simulink library "Renault_Library"  (Simulink 9.2)
settings: Solver=ode3  StartTime=0.0  StopTime=10.0  FixedStep=0.020  RelTol=1e-3  EnableMultiTasking=on
undefined symbols (not defined in the file or in its folder): ABC, AssystemProposal, b, chkval, CoeffA_C, CoeffB_C, CoeffMean_C, ConfTime_C, DiagDelay_C, DividePercent, FailureConfActive_C, FallingEdgeDuration_C, HighLevelThresholdVoltage_C, HighLevelVoltage_C, HighLevelVoltageTunable_C, InitValue_C, InputVoltRangeMax_C, InputVoltRangeMin_C, LowActiveContact_C, LowLevelThresholdVoltage_C, LowLevelVoltage_C, MaxOperatingVoltage_C, MinOperatingVoltage_C, mV2V_conversion, OUT1, OutOfRangeHigh_C, OutOfRangeLow_C, PinSupplySaturationMax, PinSupplySaturationMin, Res1Value_C, Res2Value_C, Res3Value_C, RisingEdgeDuration_C, RSA_CANSignalFailMonitoring, SampleTime_C, service14Table, service19Table, service22Table, service24Table, service28Table, service2ETable, service2FTable, service31Table, service87Table, SizeXStateDID2FTable, SizeXTable14, SizeXTable19, SizeXTable22, SizeXTable24, SizeXTable28, SizeXTable2E, SizeXTable2F, SizeXTable31, SizeXTable87, SizeYStateDID2FTable, SizeYTable14, SizeYTable19, SizeYTable22, SizeYTable24, SizeYTable28 ... (+22)
undefined symbols (not defined in the file): BatteryVoltage_ActivationThreshold_C, FrameStatus_AbsentValue_C, FrameStatus_ConfAbsentValue_C, MemThreshold_C, NAMEOFTHEMODEL_SAMPLE_TIME_CST, NbAbs1_C, NbConfAbs1_C, NbConfPres1_C, NbPres1_C, NOMDUSIGNAL_FaultConfTime_C, NOMDUSIGNAL_FaultRehabTime_C, NOMDUSIGNAL_switchvalue_C, NOMDUSIGNAL_unavailablevalue_C, PeriodFrame1_C, ResetTime_C, WakeUpTime_C
libraries: $bdroot x112, simulink x75
(part 3 of 32)

== system Renault_Library/Complex blocks/Signal Routing ==
  note: Signal Routing
  note: /!\         DO NOT USE THIS RED BLOCKS ANYMORE          /!\
      |
      |
      |
      |
      |
      |
      |
      |
      |
      |
      |
      |
      |
      |
      |
      |
      |
      |
      |
      |
      |
      |
      |
      |
      |
      |
      |
      | .
  RSA_DataTypeDuplicate : Subsystem  Tag=[LIB]  mask(RSA_DataTypeDuplicate){NoIn=3}
      mask prompts: NoIn "No Of Inputs"
      mask help:
      | Force all inputs to have the exact same data type.
      |
      | Version : 01.02
      settings: AttributesFormatString=%<MaskType> : Ver : 01.02\nNo Of Inputs %<NoIn>  CopyFcn=set(gcbh,'linkstatus','none');
      mask Help: eval(['open([','''\\F-khepri\shared\DIM-DCMAP-CGMP\66161\Shared_MatLab\Tools_DCMAP\Common_Library_MBDR\Library\Common_Library\Help\RLV_RSA_DataTypeDuplicate_Spec_V1.02.pdf''','])'])
      mask Callback NoIn:
      | f= str2num(get_param(gcb, 'NoIn'));
      | gcb;
      | d1=find_system(gcb,'LookUnderMasks','all','BlockType','Inport');
      | set_param([gcb '/Mux'],'Inputs',num2str(f));
      | if f ~= length(d1)
      | for i = 1:length(d1)
      |     b=i*50;
      |     a=150;
      |     hanblk=get_param(d1{i},'handle');
      |     blkDet=get(hanblk);
      |     pos=blkDet.PortConnectivity(1).Position;
      |     delete_line(gcb,pos);
      | delete_block(d1{i});
      | end
      | 
      | for i = 1:f
      |     b=i*50;
      |     a=150;
      |     append = num2str(i);
      |     append=strcat('/In',append);
      | 	PortName = strcat(gcb,append);
      | %     PortNameTer = strcat(PortName,'Ter');
      |     add_block('simulink/Ports & Subsystems/In1',PortName,'Position',[a-60 b-5 a-40 b+8])
      |     set_param(PortName,'ShowName','On')
      |     set_param(PortName, 'AttributesFormatString','Type : %<OutDataType>\nScaling : %<OutScaling>')
      |     set_param(PortName, 'Backgroundcolor','green');
      |     add_line(gcb,['In' num2str(i) '/1'],['Mux/' num2str(i)],'Autorouting','On');
      | %     add_block('simulink/Sinks/Terminator',PortNameTer,'Position',[a+50-60 b-5 a+50-40 b+5])
      | %     hanblk=get_param(PortName,'handle');
      | %     blkDet=get(hanblk);
      | %     pos=blkDet.PortConnectivity(1).Position;
      | %     hanblk=get_param(PortNameTer,'handle');
      | %     blkDet=get(hanblk);
      | %     pos1=blkDet.PortConnectivity(1).Position;
      | %     add_line(gcb,[pos;pos1])
      | end
      | end
      mask Display: disp('Same\n DT')
  RSA_DataTypeDuplicate_v01_03 : Subsystem  Tag=[LIB]  mask(RSA_DataTypeDuplicate){NoIn=3}
      mask prompts: NoIn "No Of Inputs"
      mask help:
      | Force all inputs to have the exact same data type.
      |
      | Version : 01.03
      mask init:
      | f= str2num(get_param(gcb, 'NoIn'));
      | gcb;
      | d1=find_system(gcb,'LookUnderMasks','all','Followlinks','on','BlockType','Inport');
      | set_param([gcb '/Mux'],'Inputs',num2str(f));
      | if f ~= length(d1)
      | for i = 1:length(d1)
      |     b=i*50;
      |     a=150;
      |     hanblk=get_param(d1{i},'handle');
      |     blkDet=get(hanblk);
      |     pos=blkDet.PortConnectivity(1).Position;
      |     delete_line(gcb,pos);
      | delete_block(d1{i});
      | end
      | 
      | for i = 1:f
      |     b=i*50;
      |     a=150;
      |     append = num2str(i);
      |     append=strcat('/In',append);
      | 	PortName = strcat(gcb,append);
      |     add_block('built-in/Inport',PortName,'Position',[a-60 b-5 a-40 b+8])
      |     set_param(PortName,'ShowName','On')
      |     set_param(PortName, 'Backgroundcolor','green'); 
      |     add_line(gcb,['In' num2str(i) '/1'],['Mux/' num2str(i)],'Autorouting','On');
      | end
      | end
      settings: AttributesFormatString=%<MaskType> : Ver : 01.03\nNo Of Inputs %<NoIn>
      mask Help: eval(['open([','''\\F-khepri\shared\DIM-DCMAP-CGMP\66161\Shared_MatLab\Tools_DCMAP\Common_Library_MBDR\Library\Common_Library\Help\RLV_RSA_DataTypeDuplicate_Spec_V1.03.pdf''','])'])
      mask Display: disp('Same\n DT')
  RSA_SlopeSwitch2Inputs : Subsystem  Tag=[LIB]  mask(RSA_SlopeSwitch2Inputs){st=0.1}
      mask prompts: st "Sample Time used for calculation, should not be inherited (-1)."
      mask help:
      | This block is a Multiple Port Slope Switch.
      | Depending on the value of input Switch_Cmd (from 0), the block outputs the corresponding input signal.
      | For Switch_Cmd = 0, Output is In0, for Switch_Cmd = 1, Output is In1, and so on...
      |
      | Nevertheless, for each switch between two input signals, a slope is activated from the first input signal to the second input signal.
      | The value of this slope is given by Pos_Slope if the input signal to reach is over the initial input signal and by Neg_Slope if the input signal to reach is under.
      | Pos_Slope and Neg_Slope are respectively the positive and negative slope parameters per second.
      |
      | The output Slope_On indicates if a transition is active (Slope_On at true) or not (Slope_On at false).
      |
      | In order to avoid the overflow, the maximum/ minimun range for the inputs Pos_Slope and Neg_Slope shall be choosen as:
      | max range(Pos_Slope)=( max range(data type(In)) – max(In));
      | min range(Neg_Slope)=( min range(data type(Input)) – min(Input));
      |
      | Version : 01.02
      mask init:
      | SS2ININI = 0;
      | STHCMD = 1;
      settings: AttributesFormatString=%<MaskType> : Ver : 01.02
      mask Help: eval(['open([','''\\F-khepri\shared\DIM-DCMAP-CGMP\66161\Shared_MatLab\Tools_DCMAP\Common_Library_MBDR\Library\Common_Library\Help\RLV_RSA_SlopeSwitch2Inputs_Spec_V1.02.pdf''','])'])
      mask Display:
      | color ('blue')
      | disp('SLOPE SWITCH\n\n 2 INPUTS')

== system Renault_Library/Complex blocks/Timers & Counters ==
  note: Timer & Counter
  note: /!\         DO NOT USE THIS RED BLOCKS ANYMORE               /!\
      |
      |
      |
      |
      |
      |
      |
      |
      |
      |
      |
      |
      |
      |
      |
      |
      |
      |
      |
      |
      |
      |
      |
      |
      |
      |
      |
      |
      |
      |
      |
      |
      |
      |
      |
      |
      |
      |
      |
      |
      |
      |
      |
      |
      |
      |
      |
      |
      |
      |
      |
      |
      |
      |
      |
      |
      |
      |
      |
      |
      |
      |
      |
      | .
  RSA_IncAndDecCounter : Subsystem  Tag=[LIB]  mask(RSA_IncAndDecCounter){}
      mask help:
      | Initially the value of “INIT_COUNTER” is set in the “COUNTER_OUTPUT” as initial value. If the “INC_COUNTER” is 1,
      | “COUNTER_OUTPUT” increases with the value of the “COUNTER_ STEP”. If the “DEC_COUNTER” is 1 and “INC_COUNTER” is 0,
      | the “COUNTER_OUTPUT” decreases with the value of the “COUNTER_ STEP”.
      |
      | The “COUNTER_OUTPUT” stops increasing or decreasing when it reaches to "MAX_COUNTER" and " MIN_COUNTER ".
      | If the “RESET_COUNTER” is 1, “COUNTER_OUTPUT” is reset to the value zero.
      |
      | Version : 01.00
      settings: AttributesFormatString=%<MaskType> : Ver : 01.00
      mask Help: eval(['open([','''\\F-khepri\shared\DIM-DCMAP-CGMP\66161\Shared_MatLab\Tools_DCMAP\Common_Library_MBDR\Library\Common_Library\Help\RLV_RSA_IncAndDecCounter_Spec_V1.00.pdf''','])'])
  RSA_IncAndDecCounter_V01_01 : Subsystem  Tag=[LIB]  mask(RSA_IncAndDecCounter){}
      mask help:
      | Initially the value of “INIT_COUNTER” is set in the “COUNTER_OUTPUT” as initial value. If the “INC_COUNTER” is 1,
      | “COUNTER_OUTPUT” increases with the value of the “COUNTER_ STEP”. If the “DEC_COUNTER” is 1 and “INC_COUNTER” is 0,
      | the “COUNTER_OUTPUT” decreases with the value of the “COUNTER_ STEP”.
      |
      | The “COUNTER_OUTPUT” stops increasing or decreasing when it reaches to "MAX_COUNTER" and " MIN_COUNTER ".
      | If the “RESET_COUNTER” is 1, “COUNTER_OUTPUT” is reset to the value zero.
      |
      | Version : 01.01
      mask init:
      | CONS_ZERO=0;
      | CONS_ONE=1;
      | CONS_TWO=2;
      | CONS_THREE=3;
      settings: AttributesFormatString=%<MaskType> : Ver : 01.01
      mask Help: eval(['open([','''\\F-khepri\shared\DIM-DCMAP-CGMP\66161\Shared_MatLab\Tools_DCMAP\Common_Library_MBDR\Library\Common_Library\Help\RLV_RSA_IncAndDecCounter_Spec_V1.01.pdf''','])'])
  RSA_Timer : Subsystem  Tag=[LIB]  mask(RSA_Timer){timeoutinit=0, initinit=0, st=0.1}
      mask prompts: timeoutinit "Timeout output initialisation"; initinit "Init input initialisation"; st "Sample Time used for calculation, should not be inherited (-1)."
      mask help:
      | This block starts a timer and generates a timeout signal when the required duration has elapsed.
      | The duration in seconds is set on rising edge 'Init' signal  and counting phase begins.
      | Output 'Timer' gives the remaining time in seconds, that must pass before generating the 'Timeout' signal.
      | If the 'Init' signal is true after the power supply, and if the timer must start, set init input initialisation to 0, else set it to 1.
      | If the 'Timeout' output must start at true, set timeout output initialisation to 1, else set it to 0.
      |
      | Version : 01.03
      mask init:
      | TIM1INI = 0;
      | Least_value = 0.000000001;
      settings: AttributesFormatString=%<MaskType> : Ver : 01.03\nTimeout output initialisation=%<timeoutinit>\nInit input initialisation=%<initinit>
      mask Help: eval(['open([','''\\F-khepri\shared\DIM-DCMAP-CGMP\66161\Shared_MatLab\Tools_DCMAP\Common_Library_MBDR\Library\Common_Library\Help\RLV_RSA_Timer_Spec_V1.03.pdf''','])'])
      mask Display:
      | plot([0.1 0.15 0.15 0.2 0.2 0.35],[0.67 0.67 0.9 0.9 0.67 0.67]);
      | plot([0.45 0.5 0.5 0.7 0.9],[0.05 0.05 0.3 0.05 0.05])
      | plot([0.45 0.7 0.7 0.9],[0.67 0.67 0.9 0.9])
  RSA_Timer1 : Subsystem  Tag=[LIB]  mask(RSA_Timer1){timeoutinit=0, initinit=0}
      mask prompts: timeoutinit "Timeout output initialisation"; initinit "Init input initialisation"
      mask help:
      | The function of the timer block is to provide "Timeout" signal after the set "Duration" is elapsed by "Timestep" when rising triggered transition by "Init" flag at input. The output value of "Duration" is also available at "Timer" output signal according to elapsed time as set via "Timestep" input signal.
      |
      | Version : 01.03
      mask init:
      | TIM1INI = 0;
      settings: AttributesFormatString=%<MaskType> : Ver : 01.03\nTimeout output initialisation=%<timeoutinit>\nInit input initialisation=%<initinit>
      mask Help: eval(['open([','''\\F-khepri\shared\DIM-DCMAP-CGMP\66161\Shared_MatLab\Tools_DCMAP\Common_Library_MBDR\Library\Common_Library\Help\RLV_RSA_Timer1_Spec_V1.03.pdf''','])'])
      mask Display:
      | plot([0.1 0.15 0.15 0.2 0.2 0.35],[0.67 0.67 0.9 0.9 0.67 0.67]);
      | plot([0.45 0.5 0.5 0.7 0.9],[0.05 0.05 0.3 0.05 0.05])
      | plot([0.45 0.7 0.7 0.9],[0.67 0.67 0.9 0.9])
  RSA_Timer1_v01_04 : Subsystem  Tag=[LIB]  mask(RSA_Timer1){timeoutinit=0, initinit=0}
      mask prompts: timeoutinit "Timeout output initialisation"; initinit "Init input initialisation"
      mask help:
      | The function of the timer block is to provide "Timeout" signal after the set "Duration" is elapsed by "Timestep" when rising triggered transition by "Init" flag at input. The output value of "Duration" is also available at "Timer" output signal according to elapsed time as set via "Timestep" input signal.
      |
      | Version : 01.04
      mask init:
      | TIM1INI = 0;
      settings: AttributesFormatString=%<MaskType> : Ver : 01.04\nTimeout output initialisation=%<timeoutinit>\nInit input initialisation=%<initinit>
      mask Help: eval(['open([','''\\F-khepri\shared\DIM-DCMAP-CGMP\66161\Shared_MatLab\Tools_DCMAP\Common_Library_MBDR\Library\Common_Library\Help\RLV_RSA_Timer1_Spec_V1.04.pdf''','])'])
      mask Display:
      | plot([0.1 0.15 0.15 0.2 0.2 0.35],[0.67 0.67 0.9 0.9 0.67 0.67]);
      | plot([0.45 0.5 0.5 0.7 0.9],[0.05 0.05 0.3 0.05 0.05])
      | plot([0.45 0.7 0.7 0.9],[0.67 0.67 0.9 0.9])
  RSA_Timer1_v01_05 : Subsystem  Tag=[LIB]  mask(RSA_Timer1){timeoutinit=0, initinit=0}
      mask prompts: timeoutinit "Timeout output initialisation"; initinit "Init input initialisation"
      mask help:
      | The function of the timer block is to provide "Timeout" signal after the set "Duration" is elapsed by "Timestep" when rising triggered transition by "Init" flag at input. The output value of "Duration" is also available at "Timer" output signal according to elapsed time as set via "Timestep" input signal.
      |
      | Note:
      | The type of the input "Duration" should not be inherited. The type should be mentioned in the input "Duration"
      |
      | Version : 01.05
      mask init:
      | TIM1INI = 0;
      settings: AttributesFormatString=%<MaskType> : Ver : 01.05\nTimeout output initialisation=%<timeoutinit>\nInit input initialisation=%<initinit>
      mask Help: eval(['open([','''\\F-khepri\shared\DIM-DCMAP-CGMP\66161\Shared_MatLab\Tools_DCMAP\Common_Library_MBDR\Library\Common_Library\Help\RLV_RSA_Timer1_Spec_V1.05.pdf''','])'])
      mask Display:
      | plot([0.1 0.15 0.15 0.2 0.2 0.35],[0.67 0.67 0.9 0.9 0.67 0.67]);
      | plot([0.45 0.5 0.5 0.7 0.9],[0.05 0.05 0.3 0.05 0.05])
      | plot([0.45 0.7 0.7 0.9],[0.67 0.67 0.9 0.9])
  RSA_Timer1_v01_06 : Subsystem  Tag=[LIB]  mask(RSA_Timer1){timeoutinit=0, initinit=0}
      mask prompts: timeoutinit "Timeout output initialisation"; initinit "Init input initialisation"
      mask help:
      | When the input2 of the block ("Init") is rising to 1, the following will be happening
      |     1. The output ("Timesout") will be Rising to 1 (TRUE) after a Delay (Duration/Timestep).
      |     2. The output "Timer" will be set with "Duration" Value and start Decrementing by SampleTime.
      |
      | When the input3 of the block ("Timestep") is falling to 0, the following will be happening
      |     1. Block will be reset to Initial State (Timeout = 0 and Timer = 0)
      |
      | Initializations:
      | - Output initialization = beginning state of Output signal.
      | - Input initialization = Used to determine rising or falling edge at the first simulation step.
      |     0 - Block will detect Edge Rising at the first simulation step itself
      |     1 - Block will detect Edge Rising at the first simulation step
      |
      | Warning: Please note that TotalDelay = (Duration/Timestep)
      |
      | Note:
      | 1. Negative Values are not allowed as an inputs (Duration,Timestep) to the block
      | 2. The Block's inputs (Duration,Timestep) must have Same Datatype
      | 3. The Block's inputs (Duration,Timestep) should not be Zero value when there is a Rising Edge of input (Init)
      |
      | Version : 01.06
      mask init:
      | TIM1INI = 0;
      | Boosting_Decimal=1000;
      | Boosting_Whole=1;
      | CONS_ONE=1;
      | CONS_ZERO=0;
      settings: AttributesFormatString=%<MaskType> : Ver : 01.06\nTimeout output initialisation=%<timeoutinit>\nInit input initialisation=%<initinit>
      mask Help: eval(['open([','''\\F-khepri\shared\DIM-DCMAP-CGMP\66161\Shared_MatLab\Tools_DCMAP\Common_Library_MBDR\Library\Common_Library\Help\RLV_RSA_Timer1_Spec_V1.06.pdf''','])'])
      mask Display:
      | plot([0.1 0.15 0.15 0.2 0.2 0.35],[0.67 0.67 0.9 0.9 0.67 0.67]);
      | plot([0.45 0.5 0.5 0.7 0.9],[0.05 0.05 0.3 0.05 0.05])
      | plot([0.45 0.7 0.7 0.9],[0.67 0.67 0.9 0.9])
  RSA_Timer_Conversion : Subsystem  Tag=[LIB]  mask(RSA_Timer_Conversion){SAMPLE_TIME_CST=NAMEOFTHEMODEL_SAMPLE_TIME_CST}
      mask prompts: SAMPLE_TIME_CST "Sample time :"
      mask help:
      | Timer Conversion block is to adapt a calibration value to output depending on a constant in block mask.
      |
      | Note:
      | The sample time constant "MODEL_NAME_SAMPLE_TIME_CST" should be defined in the workspace and the value must not be 0 or -1.
      |
      | Version : 01.00
      mask init:
      | ZERO_CST = 0;
      settings: AttributesFormatString=%<MaskType> : Ver : 01.00
      mask Help: eval(['open([','''\\F-khepri\shared\DIM-DCMAP-CGMP\66161\Shared_MatLab\Tools_DCMAP\Common_Library_MBDR\Library\Common_Library\Help\RLV_RSA_Timer_Conversion_Spec_V1.00.pdf''','])'])
  RSA_Timer_Conversion1 : Subsystem  Tag=[LIB]  mask(RSA_Timer_Conversion){SAMPLE_TIME_CST=NAMEOFTHEMODEL_SAMPLE_TIME_CST}
      mask prompts: SAMPLE_TIME_CST "Sample time :"
      mask help:
      | Timer Conversion block is to adapt a calibration value to output depending on a constant in block mask.
      |
      | Note:
      | 1) The sample time constant "MODEL_NAME_SAMPLE_TIME_CST" should be defined in the workspace and the value must not be 0 or -1.
      | 2) If the sample time is zero, then input is passed without any conversion.
      | 3) If the Sample Time in the Model where the Block is used, is not given any –ve or Zero Value, then this will lead to Model Coverage drops
      |
      | Version : 01.02
      mask init:
      | ZERO_CST = 0;
      settings: AttributesFormatString=|
      | %<MaskType> : Ver : 01.02
      | Sample Time : %<SAMPLE_TIME_CST>
      mask Help: eval(['open([','''\\F-khepri\shared\DIM-DCMAP-CGMP\66161\Shared_MatLab\Tools_DCMAP\Common_Library_MBDR\Library\Common_Library\Help\RLV_RSA_Timer_Conversion_Spec_V1.02.pdf''','])'])
  RSA_Timer_Conversion_v01_01 : Subsystem  Tag=[LIB]  mask(RSA_Timer_Conversion){SAMPLE_TIME_CST=NAMEOFTHEMODEL_SAMPLE_TIME_CST}
      mask prompts: SAMPLE_TIME_CST "Sample time :"
      mask help:
      | Timer Conversion block is to adapt a calibration value to output depending on a constant in block mask.
      |
      | Note:
      | The sample time constant "MODEL_NAME_SAMPLE_TIME_CST" shuold be defined in the workspace and the value must not be 0 or -1.
      |
      | Version : 01.01
      mask init:
      | ZERO_CST = 0;
      settings: AttributesFormatString=%<MaskType> : Ver : 01.01
      mask Help: eval(['open([','''\\F-khepri\shared\DIM-DCMAP-CGMP\66161\Shared_MatLab\Tools_DCMAP\Common_Library_MBDR\Library\Common_Library\Help\RLV_RSA_Timer_Conversion_Spec_V1.01.pdf''','])'])
  RSA_Timer_v01_04 : Subsystem  Tag=[LIB]  mask(RSA_Timer){timeoutinit=0, initinit=0, st=0.1}
      mask prompts: timeoutinit "Timeout output initialisation"; initinit "Init input initialisation"; st "Sample Time used for calculation, should not be inherited (-1)."
      mask help:
      | This block starts a timer and generates a timeout signal when the required duration has elapsed.
      | The duration in seconds is set on rising edge 'Init' signal  and counting phase begins.
      | Output 'Timer' gives the remaining time in seconds, that must pass before generating the 'Timeout' signal.
      | If the 'Init' signal is true after the power supply, and if the timer must start, set init input initialisation to 0, else set it to 1.
      | If the 'Timeout' output must start at true, set timeout output initialisation to 1, else set it to 0.
      |
      | Version : 01.04
      mask init:
      | TIM1INI = 0;
      | Least_value = 0.000000001;
      settings: AttributesFormatString=%<MaskType> : Ver : 01.04\nTimeout output initialisation=%<timeoutinit>\nInit input initialisation=%<initinit>
      mask Help: eval(['open([','''\\F-khepri\shared\DIM-DCMAP-CGMP\66161\Shared_MatLab\Tools_DCMAP\Common_Library_MBDR\Library\Common_Library\Help\RLV_RSA_Timer_Spec_V1.04.pdf''','])'])
      mask Display:
      | plot([0.1 0.15 0.15 0.2 0.2 0.35],[0.67 0.67 0.9 0.9 0.67 0.67]);
      | plot([0.45 0.5 0.5 0.7 0.9],[0.05 0.05 0.3 0.05 0.05])
      | plot([0.45 0.7 0.7 0.9],[0.67 0.67 0.9 0.9])
  RSA_Timer_v01_05 : Subsystem  Tag=[LIB]  mask(RSA_Timer){timeoutinit=0, initinit=0, st=1}
      mask prompts: timeoutinit "Timeout output initialisation"; initinit "Init input initialisation"; st "Sample Time used for calculation, should not be inherited (-1)."
      mask help:
      | This block starts a timer and generates a timeout signal when the required duration has elapsed.
      | The duration in seconds is set on rising edge 'Init' signal  and counting phase begins.
      | Output 'Timer' gives the remaining time in seconds, that must pass before generating the 'Timeout' signal.
      | If the 'Init' signal is true after the power supply, and if the timer must start, set init input initialisation to 0, else set it to 1.
      | If the 'Timeout' output must start at true, set timeout output initialisation to 1, else set it to 0.
      |
      | Note:
      | The type of the input "Duration" should not be inherited from anywhere, it should be mentioned the type of input.
      |
      | Version : 01.05
      mask init:
      | TIM1INI = 0;
      | Least_value = 0.000000001;
      settings: AttributesFormatString=%<MaskType> : Ver : 01.05\nTimeout output initialisation=%<timeoutinit>\nInit input initialisation=%<initinit>
      mask Help: eval(['open([','''\\F-khepri\shared\DIM-DCMAP-CGMP\66161\Shared_MatLab\Tools_DCMAP\Common_Library_MBDR\Library\Common_Library\Help\RLV_RSA_Timer_Spec_V1.05.pdf''','])'])
      mask Display:
      | plot([0.1 0.15 0.15 0.2 0.2 0.35],[0.67 0.67 0.9 0.9 0.67 0.67]);
      | plot([0.45 0.5 0.5 0.7 0.9],[0.05 0.05 0.3 0.05 0.05])
      | plot([0.45 0.7 0.7 0.9],[0.67 0.67 0.9 0.9])
  RSA_Timer_v01_06 : Subsystem  Tag=[LIB]  mask(RSA_Timer){timeoutinit=0, initinit=0, st=0.01}
      mask prompts: timeoutinit "Timeout output initialisation"; initinit "Init input initialisation"; st "Sample Time used for calculation, should not be inherited (-1)."
      mask help:
      | This block starts a timer and generates a timeout signal when the required duration has elapsed.
      | The duration in seconds is set on rising edge 'Init' signal  and counting phase begins.
      | Output 'Timer' gives the remaining time in seconds, that must pass before generating the 'Timeout' signal.
      | If the 'Init' signal is true after the power supply, and if the timer must start, set init input initialisation to 0, else set it to 1.
      | If the 'Timeout' output must start at true, set timeout output initialisation to 1, else set it to 0.
      |
      | Note:
      | The type of the input "Duration" should not be inherited from anywhere, it should be mentioned the type of input.
      |
      | Version : 01.06
      mask init:
      | TIM1INI = 0;
      | Least_value = 0.000000001;
      settings: AttributesFormatString=%<MaskType> : Ver : 01.06\nTimeout output initialisation=%<timeoutinit>\nInit input initialisation=%<initinit>
      mask Help: eval(['open([','''\\F-khepri\shared\DIM-DCMAP-CGMP\66161\Shared_MatLab\Tools_DCMAP\Common_Library_MBDR\Library\Common_Library\Help\RLV_RSA_Timer_Spec_V1.06.pdf''','])'])
      mask Display:
      | plot([0.1 0.15 0.15 0.2 0.2 0.35],[0.67 0.67 0.9 0.9 0.67 0.67]);
      | plot([0.45 0.5 0.5 0.7 0.9],[0.05 0.05 0.3 0.05 0.05])
      | plot([0.45 0.7 0.7 0.9],[0.67 0.67 0.9 0.9])
  RSA_Timer_v01_07 : Subsystem  Tag=[LIB]  mask(RSA_Timer){timeoutinit=0, initinit=0, st=0.1, SampleDT=double}
      mask prompts: timeoutinit "Timeout output initialisation"; initinit "Init input initialisation"; st "Sample Time used for calculation, should not be inherited (-1)."; SampleDT "DataType for Sample Time"
      mask help:
      | When the input1 of the block ("Init") is rising to 1, the following will be happening
      |     1. The output "Timer" will be set with "Duration" Value and start Decrementing by SampleTime.
      |     2. The output ("Timeout") will be Rising to 1 (TRUE) after a Delay (Duration/SampleTime).
      |
      | When the input1 of the block ("Init") is falling to 0, the following will be happening
      |     1. Block will be reset to Initial State (delay_out = 0 and Timer =0)
      |
      | WARNING: Please note that Total Delay  = (Duration/SampleTime).
      |
      | Version : 01.07
      mask init:
      | TIM1INI = 0;
      | fix_flag = ~isempty(regexp(get(gcbh,'SampleDT'),'^single$|^double$'));
      | if fix_flag
      |     Boosting_Factor = 1000;
      |     Constant_DT = get(gcbh,'SampleDT');
      | else
      |     Boosting_Factor = 1;
      |     Constant_DT = 'uint8';
      | end
      settings: AttributesFormatString=|
      | %<MaskType> : Ver : 01.07\nTimeout output initialisation=%<timeoutinit>\nInit input initialisation=%<initinit>
      | Sample Time : %<st>
      | Sample Time DataType :%<SampleDT>
      mask Help: eval(['open([','''\\F-khepri\shared\DIM-DCMAP-CGMP\66161\Shared_MatLab\Tools_DCMAP\Common_Library_MBDR\Library\Common_Library\Help\RLV_RSA_Timer_Spec_V1.07.pdf''','])'])
      mask Display:
      | plot([0.1 0.15 0.15 0.2 0.2 0.35],[0.67 0.67 0.9 0.9 0.67 0.67]);
      | plot([0.45 0.5 0.5 0.7 0.9],[0.05 0.05 0.3 0.05 0.05])
      | plot([0.45 0.7 0.7 0.9],[0.67 0.67 0.9 0.9])
  RSA_TurnOffDelay2 : Subsystem  Tag=[LIB]  mask(RSA_TurnOffDelay2){Ts=0.1}
      mask prompts: Ts "Sample Time used for calculation, should not be inherited (-1)."
      mask help:
      | TurnOff Delay block will delay the turnoff time 'out' with respect to the given delay time 'dly'.
      |
      | Version : 01.02
      mask init:
      | INIVAL = 0;
      settings: AttributesFormatString=%<MaskType> : Ver : 01.02
      mask Help:
      | TurnOff Delay block will delay the turnoff time 'out' with respect to the given delay time 'dly'.
      | 
      | Version : 01.02
      mask Display:
      | plot([-1.05,12.05],[0.97,0.97]*11);
      | plot([0  1  1  2  2  4  4  7  7  9  9 11],...
      | 	 [10 10 8  8 10 10  8  8  8  8 10 10]);
      | plot([0  1  1  2  2  4  4  7  7  9  9 11],...
      | 	 [4  4  7  6  6  6  7  4  4  4  4  4]);
      | plot([0  1  1  2  2  4  4  7  7  9  9 11],...
      | 	 [3  3  3  3  3  3  3  3  1  1  3  3]);
      | plot(-1,0); plot(12,11);
      | text(4.5,9,'in');
      | text(4.5,5,'ctr');
      | text(4.5,2,'out');
  RSA_TurnOffDelay2_v01_03 : Subsystem  Tag=[LIB]  mask(RSA_TurnOffDelay2){Ts=0.1}
      mask prompts: Ts "Sample Time used for calculation, should not be inherited (-1)."
      mask help:
      | TurnOff Delay block will delay the turnoff time 'out' with respect to the given delay time 'dly'.
      |
      | Version : 01.03
      mask init:
      | INIVAL = 0;
      settings: AttributesFormatString=%<MaskType> : Ver : 01.03
      mask Help: eval(['open([','''\\F-khepri\shared\DIM-DCMAP-CGMP\66161\Shared_MatLab\Tools_DCMAP\Common_Library_MBDR\Library\Common_Library\Help\RLV_RSA_TurnOffDelay2_Spec_V1.03.pdf''','])'])
      mask Display:
      | plot([-1.05,12.05],[0.97,0.97]*11);
      | plot([0  1  1  2  2  4  4  7  7  9  9 11],...
      | 	 [10 10 8  8 10 10  8  8  8  8 10 10]);
      | plot([0  1  1  2  2  4  4  7  7  9  9 11],...
      | 	 [4  4  7  6  6  6  7  4  4  4  4  4]);
      | plot([0  1  1  2  2  4  4  7  7  9  9 11],...
      | 	 [3  3  3  3  3  3  3  3  1  1  3  3]);
      | plot(-1,0); plot(12,11);
      | text(4.5,9,'in');
      | text(4.5,5,'ctr');
      | text(4.5,2,'out');
  RSA_TurnOffDelay2_v01_05 : Subsystem  Tag=[LIB]  mask(RSA_TurnOffDelay2){Ts=1}
      mask prompts: Ts "Sample Time used for calculation, should not be inherited (-1)."
      mask help:
      | TurnOff Delay block will delay the turnoff time 'out' with respect to the given delay time 'dly'.
      |
      | Note:
      | The type of the input "dly" should not be inherited from anywhere, it should be defined with any type.
      | Version : 01.05
      mask init:
      | INIVAL = 0;
      settings: AttributesFormatString=%<MaskType> : Ver : 01.05
      mask Help: eval(['open([','''\\F-khepri\shared\DIM-DCMAP-CGMP\66161\Shared_MatLab\Tools_DCMAP\Common_Library_MBDR\Library\Common_Library\Help\RLV_RSA_TurnOffDelay2_Spec_V1.05.pdf''','])'])
      mask Display:
      | plot([-1.05,12.05],[0.97,0.97]*11);
      | plot([0  1  1  2  2  4  4  7  7  9  9 11],...
      | 	 [10 10 8  8 10 10  8  8  8  8 10 10]);
      | plot([0  1  1  2  2  4  4  7  7  9  9 11],...
      | 	 [4  4  7  6  6  6  7  4  4  4  4  4]);
      | plot([0  1  1  2  2  4  4  7  7  9  9 11],...
      | 	 [3  3  3  3  3  3  3  3  1  1  3  3]);
      | plot(-1,0); plot(12,11);
      | text(4.5,9,'in');
      | text(4.5,5,'ctr');
      | text(4.5,2,'out');
  RSA_TurnOffDelay2_v01_06 : Subsystem  Tag=[LIB]  mask(RSA_TurnOffDelay2){Ts=0.1, SampleDT=double}
      mask prompts: Ts "Sample Time used for calculation, should not be inherited (-1)."; SampleDT "DataType for Sample Time"
      mask help:
      | When the input1 of the block ("delay_flag") is falling to 0, the following will be happening
      |     1. The output "Ctr" will be set with "Dly" Value and start Decrementing by SampleTime.
      |     2. The output ("delay_out") will be falling to 0 (FALSE) after a Delay (Dly/SampleTime).
      |
      | When the input1 of the block ("delay_flag") is rising to 1, the following will be happening
      |     1. Block will be reset to Initial State (delay_out = 1 and Ctr =0)
      |
      | WARNING: Please note that Total Delay  = (Dly/SampleTime).
      |
      | NOTE:
      |     1.When fixed data types is given for input ("dly"), both the input ("dly") and sample time (Ts specified in mask) should have the same resolution.
      |     2.When fixed data types is given for input ("dly"), the sample time's data type in the mask should be set as "Uint8".
      |     3.The input "dly" should be set such that it is a multiple of sample time "Ts". Eg. If "dly" is 1 then allowed sample time (Ts) are 0.1,0.2,0.02, etc.,
      |
      | Version : 01.06
      mask init:
      | %check_non_inherited_samp(Ts);
      | INIVAL = 0;
      | fix_flag = ~isempty(regexp(get(gcbh,'SampleDT'),'^single$|^double$'));
      | if fix_flag
      |     Boosting_Factor = 1000;
      |     Constant_DT = get(gcbh,'SampleDT');
      | else
      |     Boosting_Factor = 1;
      |     Constant_DT = 'uint8';
      | end
      settings: AttributesFormatString=|
      | %<MaskType> : Ver : 01.06
      | Sample Time : %<Ts>
      | Sample Time DataType : %<SampleDT>
      mask Help: eval(['open([','''\\F-khepri\shared\DIM-DCMAP-CGMP\66161\Shared_MatLab\Tools_DCMAP\Common_Library_MBDR\Library\Common_Library\Help\RLV_RSA_TurnOffDelay2_Spec_V1.06.pdf''','])'])
      mask Display:
      | plot([-1.05,12.05],[0.97,0.97]*11);
      | plot([0  1  1  2  2  4  4  7  7  9  9 11],...
      | 	 [10 10 8  8 10 10  8  8  8  8 10 10]);
      | plot([0  1  1  2  2  4  4  7  7  9  9 11],...
      | 	 [4  4  7  6  6  6  7  4  4  4  4  4]);
      | plot([0  1  1  2  2  4  4  7  7  9  9 11],...
      | 	 [3  3  3  3  3  3  3  3  1  1  3  3]);
      | plot(-1,0); plot(12,11);
      | text(4.5,9,'dly_flag');
      | text(4.5,5,'ctr');
      | text(4.5,2,'dly_out');
  RSA_TurnOffDelay_v01_00 : Subsystem  Tag=[LIB]  mask(RSA_TurnOffDelay){outINI=TRUE, inINI=FALSE}
      mask prompts: outINI "Output initialization (false or true)"; inINI "Input initialization (false or true)"
      mask help:
      | When the input1 of the block ("signal") is falling to 0, the following will be happening
      |     1. The output "Ctr" will be set with "Dly" Value and start Decrementing by TimeStep.
      |     2. The output ("out") will be falling to 0 (FALSE) after a Delay (DelayTime/ TimeStep).
      |
      | When the input1 of the block ("signal") is rising to 1, the following will be happening
      |     1. Block will be reset to Initial State (out = 1 and Ctr =0)
      |
      | WARNING: Please note that Total Delay  = (DelayTime / TimeStep).
      | Initializations:
      | - Output initialization = beginning state of Output signal.
      |
      | - Input initialization = Used to determine falling edge at the first simulation step.
      |    1 - Block will detect Edge Falling at the first simulation step itself.
      |    0 - Block will not detect Edge Falling at the first simulation step.
      |
      | Warning: Please note that TotalDelay = (Delaytime/Timestep)
      |
      | Note:
      | 1. Negative Values are not allowed as an inputs (Delaytime,Timestep) to the block
      | 2. The Block's inputs (Delaytime,Timestep) must have Same Datatype
      | 3. The Block's inputs (Delaytime,Timestep) should not be Zero value when there is a Falling Edge of input (Signal)
      | Version: 01.00
      mask init:
      | TIM1INI = 0;
      | CONS_ONE =1;
      | CONS_ZERO=0;
      | BOOSTING_FLP=1000;
      | BOOSTING_FXP=1;
      | if inINI == 2
      |     initinit = boolean(1);
      | else
      |     initinit = boolean(0);
      | end
      | if outINI == 2
      |     timeoutinit = boolean(1);
      | else
      |     timeoutinit = boolean(0);
      | end
      | 
      | if outINI == 2
      |     set_param([gcb '/From7'],'gototag','InitOut');
      | else
      |     set_param([gcb '/From7'],'gototag','InitOut_Cond');
      | end
      settings: AttributesFormatString=%<MaskType> : Ver : 01.00\nOutput initialization=%<outINI>\nInput initialization=%<inINI>
      mask Help: eval(['open([','''\\F-khepri\shared\DIM-DCMAP-CGMP\66161\Shared_MatLab\Tools_DCMAP\Common_Library_MBDR\Library\Common_Library\Help\RLV_RSA_TurnOffDelay_Spec_V1.00.pdf''','])'])
      mask TypeOptions outINI: FALSE | TRUE
      mask TypeOptions inINI: FALSE | TRUE
      mask Display:
      | plot([-1.05,12.05],[0.97,0.97]*11);
      | plot([0  1  1  2  2  4  4  7  7  9  9 11],...
      | 	 [10 10 8  8 10 10  8  8  8  8 10 10]);
      | plot([0  1  1  2  2  4  4  7  7  9  9 11],...
      | 	 [4  4  7  6  6  6  7  4  4  4  4  4]);
      | plot([0  1  1  2  2  4  4  7  7  9  9 11],...
      | 	 [3  3  3  3  3  3  3  3  1  1  3  3]);
      | plot(-1,0); plot(12,11);
      | text(4.5,9,'dly_flag');
      | text(4.5,5,'ctr');
      | text(4.5,2,'dly_out');
  RSA_TurnOnDelay : Subsystem  Tag=[LIB]  mask(RSA_TurnOnDelay){timeoutinit=0, initinit=1}
      mask prompts: timeoutinit "Output initialization (0 or 1)"; initinit "Input initialization (0 or 1)"
      mask help:
      | The RSA_TurnOnDelay function applies a boolean input to the rising edge of the input Signal and delay time on the other input port. The output (Out) is a boolean signal changes from 0 to 1 after the specified delay time.
      |
      | Initializations:
      | - Output initialization = beginning state of Output signal.
      | - Input initialization = state of Signal at -t used to determine the presence of a rising or falling edge at the first simulation step.
      |
      | Version : 01.02
      settings: AttributesFormatString=%<MaskType> : Ver : 01.02\nOutput initialization=%<timeoutinit>\nInput initialization=%<initinit>
      mask Help: eval(['open([','''\\F-khepri\shared\DIM-DCMAP-CGMP\66161\Shared_MatLab\Tools_DCMAP\Common_Library_MBDR\Library\Common_Library\Help\RLV_RSA_TurnOnDelay_Spec_V1.02.pdf''','])'])
      mask Display:
      | plot([3 3.5 3.5 4 4 5 5 7.5 7.5 8.5], [5 5 6 6 5 5 6 6 5 5]);
      | plot([3 6.5 6.5 7.5 7.5 8.5], [2 2 3 3 2 2]);
      | plot([3 4.5], [5 6]);
      | plot([3 4.5], [6 5]);
      | text(5,0.4, 'Duration');
      | plot([5 6.5], [1 1]);
      | plot([5.2 5 5.2], [0.8 1 1.2]);
      | plot([6.3 6.5 6.3], [0.8 1 1.2]);
      | plot([5 5],[4.7 1.3]);
      | plot([6.5 6.5],[1.8 1.3]);
      | plot([7.5 7.5],[4.7 3.7]);
      | plot([0], [0]);
      | plot([8], [7]);
  RSA_TurnOnDelay2 : Subsystem  Tag=[LIB]  mask(RSA_TurnOnDelay2){Ts=0.1}
      mask prompts: Ts "Sample Time used for calculation, should not be inherited (-1)."
      mask help:
      | The output follows input state with a delay to turn ON, without any delay to turn OFF
      | WARNING: dly = counting delay in seconds.
      |
      | Version : 01.02
      mask init:
      | %check_non_inherited_samp(Ts);
      | INIVAL = 0;
      settings: AttributesFormatString=%<MaskType> : Ver : 01.02
      mask Help:
      | The output follows input state with a delay to turn ON, without any delay to turn OFF
      | WARNING: dly = counting delay in seconds.
      | 
      | Version : 01.02
      mask Display:
      | plot([-1.05,12.05],[0.97,0.97]*11);
      | plot([0  1  1  2  2  4  4  7  7  9  9 11],...
      | 	 [8  8 10 10  8  8 10 10 10 10  8  8]);
      | plot([0  1  1  2  2  4  4  7  7  9  9 11],...
      | 	 [4  4  7  6  6  6  7  4  4  4  4  4]);
      | plot([0  1  1  2  2  4  4  7  7  9  9 11],...
      | 	 [1  1  1  1  1  1  1  1  3  3  1  1]);
      | plot(-1,0); plot(12,11);
      | text(4.5,9,'in');
      | text(4.5,5,'ctr');
      | text(4.5,2,'out');
  RSA_TurnOnDelay2_v01_03 : Subsystem  Tag=[LIB]  mask(RSA_TurnOnDelay2){Ts=0.1}
      mask prompts: Ts "Sample Time used for calculation, should not be inherited (-1)."
      mask help:
      | The output follows input state with a delay to turn ON, without any delay to turn OFF
      | WARNING: dly = counting delay in seconds.
      |
      | Version : 01.03
      mask init:
      | %check_non_inherited_samp(Ts);
      | INIVAL = 0;
      settings: AttributesFormatString=%<MaskType> : Ver : 01.03
      mask Help: eval(['open([','''\\F-khepri\shared\DIM-DCMAP-CGMP\66161\Shared_MatLab\Tools_DCMAP\Common_Library_MBDR\Library\Common_Library\Help\RLV_RSA_TurnOnDelay2_Spec_V1.03.pdf''','])'])
      mask Display:
      | plot([-1.05,12.05],[0.97,0.97]*11);
      | plot([0  1  1  2  2  4  4  7  7  9  9 11],...
      | 	 [8  8 10 10  8  8 10 10 10 10  8  8]);
      | plot([0  1  1  2  2  4  4  7  7  9  9 11],...
      | 	 [4  4  7  6  6  6  7  4  4  4  4  4]);
      | plot([0  1  1  2  2  4  4  7  7  9  9 11],...
      | 	 [1  1  1  1  1  1  1  1  3  3  1  1]);
      | plot(-1,0); plot(12,11);
      | text(4.5,9,'in');
      | text(4.5,5,'ctr');
      | text(4.5,2,'out');
  RSA_TurnOnDelay2_v01_04 : Subsystem  Tag=[LIB]  mask(RSA_TurnOnDelay2){Ts=0.1}
      mask prompts: Ts "Sample Time used for calculation, should not be inherited (-1)."
      mask help:
      | The output follows input state with a delay to turn ON, without any delay to turn OFF
      | WARNING: dly = counting delay in seconds.
      |
      | Note:
      | The type of the input "dly" should not be inherited from anywhere, the type should be defined.
      |
      | Version : 01.04
      mask init:
      | %check_non_inherited_samp(Ts);
      | INIVAL = 0;
      settings: AttributesFormatString=%<MaskType> : Ver : 01.04
      mask Help: eval(['open([','''\\F-khepri\shared\DIM-DCMAP-CGMP\66161\Shared_MatLab\Tools_DCMAP\Common_Library_MBDR\Library\Common_Library\Help\RLV_RSA_TurnOnDelay2_Spec_V1.04.pdf''','])'])
      mask Display:
      | plot([-1.05,12.05],[0.97,0.97]*11);
      | plot([0  1  1  2  2  4  4  7  7  9  9 11],...
      | 	 [8  8 10 10  8  8 10 10 10 10  8  8]);
      | plot([0  1  1  2  2  4  4  7  7  9  9 11],...
      | 	 [4  4  7  6  6  6  7  4  4  4  4  4]);
      | plot([0  1  1  2  2  4  4  7  7  9  9 11],...
      | 	 [1  1  1  1  1  1  1  1  3  3  1  1]);
      | plot(-1,0); plot(12,11);
      | text(4.5,9,'in');
      | text(4.5,5,'ctr');
      | text(4.5,2,'out');
  RSA_TurnOnDelay2_v01_05 : Subsystem  Tag=[LIB]  mask(RSA_TurnOnDelay2){Ts=0.01}
      mask prompts: Ts "Sample Time used for calculation, should not be inherited (-1)."
      mask help:
      | The output follows input state with a delay to turn ON, without any delay to turn OFF
      | WARNING: dly = counting delay in seconds.
      |
      | Note:
      | The type of the input "dly" should not be inherited from anywhere, the type should be defined.
      |
      | Version : 01.05
      mask init:
      | %check_non_inherited_samp(Ts);
      | INIVAL = 0;
      settings: AttributesFormatString=%<MaskType> : Ver : 01.05
      mask Help: eval(['open([','''\\F-khepri\shared\DIM-DCMAP-CGMP\66161\Shared_MatLab\Tools_DCMAP\Common_Library_MBDR\Library\Common_Library\Help\RLV_RSA_TurnOnDelay2_Spec_V1.05.pdf''','])'])
      mask Display:
      | plot([-1.05,12.05],[0.97,0.97]*11);
      | plot([0  1  1  2  2  4  4  7  7  9  9 11],...
      | 	 [8  8 10 10  8  8 10 10 10 10  8  8]);
      | plot([0  1  1  2  2  4  4  7  7  9  9 11],...
      | 	 [4  4  7  6  6  6  7  4  4  4  4  4]);
      | plot([0  1  1  2  2  4  4  7  7  9  9 11],...
      | 	 [1  1  1  1  1  1  1  1  3  3  1  1]);
      | plot(-1,0); plot(12,11);
      | text(4.5,9,'in');
      | text(4.5,5,'ctr');
      | text(4.5,2,'out');
  RSA_TurnOnDelay2_v01_06 : Subsystem  Tag=[LIB]  mask(RSA_TurnOnDelay2){Ts=0.1, SampleDT=double}
      mask prompts: Ts "Sample Time used for calculation, should not be inherited (-1)."; SampleDT "DataType for Sample Type"
      mask help:
      | When the input1 of the block ("delay_flag") is rising to 1, the following will be happening
      |     1. The output "Ctr" will be set with "Dly" Value and start Decrementing by SampleTime.
      |     2. The output ("delay_out") will be Rising to 1 (TRUE) after a Delay (Dly/SampleTime).
      |
      | When the input1 of the block ("delay_flag") is falling to 0, the following will be happening
      |     1. Block will be reset to Initial State (delay_out = 0 and Ctr =0)
      |
      | WARNING: Please note that Total Delay  = (Dly/SampleTime).
      |
      | Version : 01.06
      mask init: same as Renault_Library/Complex blocks/Timers & Counters/RSA_TurnOffDelay2_v01_06
      settings: AttributesFormatString=|
      | %<MaskType> : Ver : 01.06
      | SampleTime : %<Ts>
      | SampleTime DataType : %<SampleDT>
      mask Help: eval(['open([','''\\F-khepri\shared\DIM-DCMAP-CGMP\66161\Shared_MatLab\Tools_DCMAP\Common_Library_MBDR\Library\Common_Library\Help\RLV_RSA_TurnOnDelay2_Spec_V1.06.pdf''','])'])
      mask Display:
      | % plot([-1.05,12.05],[0.97,0.97]*11);
      | plot([0  1  1  2  2  4  4  7  7  9  9 11],...
      | 	 [8  8 10 10  8  8 10 10 10 10  8  8]);
      | plot([0  1  1  2  2  4  4  7  7  9  9 11],...
      | 	 [4  4  7  6  4  4  7  4  4  4  4  4]);
      | plot([0  1  1  2  2  4  4  7  7  9  9 11],...
      | 	 [1  1  1  1  1  1  1  1  3  3  1  1]);
      | plot(-1,0); plot(12,11);
      | text(4.5,9,'dly_flg');
      | text(4.5,5,'ctr');
      | text(4.5,2,'dly_out');
  RSA_TurnOnDelay_v01_03 : Subsystem  Tag=[LIB]  mask(RSA_TurnOnDelay){timeoutinit=0, initinit=0}
      mask prompts: timeoutinit "Output initialization (0 or 1)"; initinit "Input initialization (0 or 1)"
      mask help:
      | The RSA_TurnOnDelay function applies a boolean input to the rising edge of the input Signal and delay time on the other input port. The output (Out) is a boolean signal changes from 0 to 1 after the specified delay time.
      |
      | Initializations:
      | - Output initialization = beginning state of Output signal.
      | - Input initialization = state of Signal at -t used to determine the presence of a rising or falling edge at the first simulation step.
      |
      | Version : 01.03
      settings: AttributesFormatString=%<MaskType> : Ver : 01.03\nOutput initialization=%<timeoutinit>\nInput initialization=%<initinit>
      mask Help: eval(['open([','''\\F-khepri\shared\DIM-DCMAP-CGMP\66161\Shared_MatLab\Tools_DCMAP\Common_Library_MBDR\Library\Common_Library\Help\RLV_RSA_TurnOnDelay_Spec_V1.03.pdf''','])'])
      mask Display:
      | plot([3 3.5 3.5 4 4 5 5 7.5 7.5 8.5], [5 5 6 6 5 5 6 6 5 5]);
      | plot([3 6.5 6.5 7.5 7.5 8.5], [2 2 3 3 2 2]);
      | plot([3 4.5], [5 6]);
      | plot([3 4.5], [6 5]);
      | text(5,0.4, 'Duration');
      | plot([5 6.5], [1 1]);
      | plot([5.2 5 5.2], [0.8 1 1.2]);
      | plot([6.3 6.5 6.3], [0.8 1 1.2]);
      | plot([5 5],[4.7 1.3]);
      | plot([6.5 6.5],[1.8 1.3]);
      | plot([7.5 7.5],[4.7 3.7]);
      | plot([0], [0]);
      | plot([8], [7]);
  RSA_TurnOnDelay_v01_04 : Subsystem  Tag=[LIB]  mask(RSA_TurnOnDelay){timeoutinit=0, initinit=0}
      mask prompts: timeoutinit "Output initialization (0 or 1)"; initinit "Input initialization (0 or 1)"
      mask help:
      | The RSA_TurnOnDelay function applies a boolean input to the rising edge of the input Signal and delay time on the other input port. The output (Out) is a boolean signal changes from 0 to 1 after the specified delay time.
      |
      | Initializations:
      | - Output initialization = beginning state of Output signal.
      | - Input initialization = state of Signal at -t used to determine the presence of a rising or falling edge at the first simulation step.
      |
      | Note:
      | The type of the input "Timestep" should be mentioned and it should not be back propagated from anywhere.
      |
      | Version : 01.04
      mask init:
      | TIM1INI = 0;
      settings: AttributesFormatString=%<MaskType> : Ver : 01.04\nOutput initialization=%<timeoutinit>\nInput initialization=%<initinit>
      mask Help: eval(['open([','''\\F-khepri\shared\DIM-DCMAP-CGMP\66161\Shared_MatLab\Tools_DCMAP\Common_Library_MBDR\Library\Common_Library\Help\RLV_RSA_TurnOnDelay_Spec_V1.04.pdf''','])'])
      mask Display:
      | plot([3 3.5 3.5 4 4 5 5 7.5 7.5 8.5], [5 5 6 6 5 5 6 6 5 5]);
      | plot([3 6.5 6.5 7.5 7.5 8.5], [2 2 3 3 2 2]);
      | plot([3 4.5], [5 6]);
      | plot([3 4.5], [6 5]);
      | text(5,0.4, 'Duration');
      | plot([5 6.5], [1 1]);
      | plot([5.2 5 5.2], [0.8 1 1.2]);
      | plot([6.3 6.5 6.3], [0.8 1 1.2]);
      | plot([5 5],[4.7 1.3]);
      | plot([6.5 6.5],[1.8 1.3]);
      | plot([7.5 7.5],[4.7 3.7]);
      | plot([0], [0]);
      | plot([8], [7]);
  RSA_TurnOnDelay_v01_05 : Subsystem  Tag=[LIB]  mask(RSA_TurnOnDelay){timeoutinit=0, initinit=0}
      mask prompts: timeoutinit "Output initialization (0 or 1)"; initinit "Input initialization (0 or 1)"
      mask help:
      | When the input2 of the block ("Signal") is rising to 1, the following will be happening
      |     1. The output ("Out") will be Rising to 1 (TRUE) after a Delay (Delaytime/Timestep).
      |
      | When the input3 of the block ("Timestep") is falling to 0, the following will be happening
      |     1. Block will be reset to Initial State (Out = 0)
      |
      | Initializations:
      | - Output initialization = beginning state of Output signal.
      | - Input initialization = Used to determine rising or falling edge at the first simulation step.
      |     0 - Block will detect Edge Rising at the first simulation step itself
      |     1 - Block will detect Edge Rising at the first simulation step
      |
      | Warning: Please note that TotalDelay = (Delaytime/Timestep)
      |
      | Note:
      | 1. Negative Values are not allowed as an inputs (Delaytime,Timestep) to the block
      | 2. The Block's inputs (Delaytime,Timestep) must have Same Datatype
      | 3. The Block's inputs (Delaytime,Timestep) should not be Zero value when there is a Rising Edge of input (Signal)
      |
      | Version : 01.05
      mask init:
      | TIM1INI = 0;
      | CONS_ONE =1;
      | CONS_ZERO=0;
      | BOOSTING_FLP=1000;
      | BOOSTING_FXP=1;
      settings: AttributesFormatString=%<MaskType> : Ver : 01.05\nOutput initialization=%<timeoutinit>\nInput initialization=%<initinit>
      mask Help: eval(['open([','''\\F-khepri\shared\DIM-DCMAP-CGMP\66161\Shared_MatLab\Tools_DCMAP\Common_Library_MBDR\Library\Common_Library\Help\RLV_RSA_TurnOnDelay_Spec_V1.05.pdf''','])'])
      mask Display:
      | plot([3 3.5 3.5 4 4 5 5 7.5 7.5 8.5], [5 5 6 6 5 5 6 6 5 5]);
      | plot([3 6.5 6.5 7.5 7.5 8.5], [2 2 3 3 2 2]);
      | plot([3 4.5], [5 6]);
      | plot([3 4.5], [6 5]);
      | text(5,0.4, 'Duration');
      | plot([5 6.5], [1 1]);
      | plot([5.2 5 5.2], [0.8 1 1.2]);
      | plot([6.3 6.5 6.3], [0.8 1 1.2]);
      | plot([5 5],[4.7 1.3]);
      | plot([6.5 6.5],[1.8 1.3]);
      | plot([7.5 7.5],[4.7 3.7]);
      | plot([0], [0]);
      | plot([8], [7]);
